simulink model "CCMVgaMotor"  (R2022b) (continued)
== system CCMVgaMotor/CCMVgaMotor_Runnable_sys == (continued)
      settings: EnsureOutportIsVirtual=on
  VentnMotSaveData_VentnMotSaveData_write : Outport  Port=15
      settings: EnsureOutportIsVirtual=on
  VentnMotSaveData2_VentnMotSaveData2_write : Outport  Port=16
      settings: EnsureOutportIsVirtual=on
  VentnMotSpdSet_VentnMotSpdSet_write : Outport  Port=17
      settings: EnsureOutportIsVirtual=on
  VentnMotSpdSet2_VentnMotSpdSet2_write : Outport  Port=18
      settings: EnsureOutportIsVirtual=on
  VentnMotStrtPson_VentnMotStrtPson_write : Outport  Port=19
      settings: EnsureOutportIsVirtual=on
  VentnMotStrtPson2_VentnMotStrtPson2_write : Outport  Port=20
      settings: EnsureOutportIsVirtual=on
  VentnMotTargtPson_VentnMotTargtPson_write : Outport  Port=21
      settings: EnsureOutportIsVirtual=on
  VentnMotTargtPson2_VentnMotTargtPson2_write : Outport  Port=22
      settings: EnsureOutportIsVirtual=on
  VentnMotUrgcPson_VentnMotUrgcPson_write : Outport  Port=23
      settings: EnsureOutportIsVirtual=on
  VentnMotUrgcPson2_VentnMotUrgcPson2_write : Outport  Port=24
      settings: EnsureOutportIsVirtual=on
  VentnMotUrgcUnlck_VentnMotUrgcUnlck_write : Outport  Port=25
      settings: EnsureOutportIsVirtual=on
  VentnMotUrgcUnlck2_VentnMotUrgcUnlck2_write : Outport  Port=26
      settings: EnsureOutportIsVirtual=on
  wires:
  CarCfgForClimaCtrl_CarCfgForAirClng_read/1 -> Terminator_1/1
  CarCfgForClimaCtrl_CarCfgForAirClnrAdv_read/1 -> Terminator_2/1
  CarCfgForClimaCtrl_CarCfgForAirFrag_read/1 -> Terminator_3/1
  CarCfgForClimaCtrl_CarCfgForAirMtrlSnsr_read/1 -> Terminator_4/1
  CarCfgForClimaCtrl_CarCfgForAirQly_read/1 -> Terminator_5/1
  CarCfgForClimaCtrl_CarCfgForAmntOfSeat_read/1 -> Terminator_6/1
  CarCfgForClimaCtrl_CarCfgForAuxWtrPmp_read/1 -> Terminator_7/1
  CarCfgForClimaCtrl_CarCfgForBypVlvInHvac_read/1 -> Terminator_8/1
  CarCfgForClimaCtrl_CarCfgForClsVlvInHvac_read/1 -> Terminator_9/1
  CarCfgForClimaCtrl_CarCfgForCmprAcTyp_read/1 -> Terminator_10/1
  CarCfgForClimaCtrl_CarCfgForCmptmtClng_read/1 -> Terminator_11/1
  CarCfgForClimaCtrl_CarCfgForElecVentAdjmt_read/1 -> Terminator_12/1
  CarCfgForClimaCtrl_CarCfgForFlcTyp_read/1 -> Terminator_13/1
  CarCfgForClimaCtrl_CarCfgForFoldCSD_read/1 -> Terminator_14/1
  CarCfgForClimaCtrl_CarCfgForFuTyp_read/1 -> Terminator_15/1
  CarCfgForClimaCtrl_CarCfgForGlassTyp_read/1 -> Terminator_16/1
  CarCfgForClimaCtrl_CarCfgForHeatPmp_read/1 -> Terminator_17/1
  CarCfgForClimaCtrl_CarCfgForHeatrDurgDrvg_read/1 -> Terminator_18/1
  CarCfgForClimaCtrl_CarCfgForHeatrTyp_read/1 -> Terminator_19/1
  CarCfgForClimaCtrl_CarCfgForHvacFilTyp_read/1 -> Terminator_20/1
  CarCfgForClimaCtrl_CarCfgForHvacFlrSnsrForT_read/1 -> Terminator_21/1
  CarCfgForClimaCtrl_CarCfgForHvacHeatrSnsrT_read/1 -> Terminator_22/1
  CarCfgForClimaCtrl_CarCfgForHvacRe_read/1 -> Terminator_23/1
  CarCfgForClimaCtrl_CarCfgForHvacTyp_read/1 -> Terminator_24/1
  CarCfgForClimaCtrl_CarCfgForLoVoltElecCfg_read/1 -> Terminator_25/1
  CarCfgForClimaCtrl_CarCfgForNavSysandIntnmap_read/1 -> Terminator_26/1
  CarCfgForClimaCtrl_CarCfgForNrOfDoor_read/1 -> Terminator_27/1
  CarCfgForClimaCtrl_CarCfgForPipeInFuTank_read/1 -> Terminator_28/1
  CarCfgForClimaCtrl_CarCfgForPreClng_read/1 -> Terminator_29/1
  CarCfgForClimaCtrl_CarCfgForPrkgCDSNotAvl_read/1 -> Terminator_30/1
  CarCfgForClimaCtrl_CarCfgForPrkgClima_read/1 -> Terminator_31/1
  CarCfgForClimaCtrl_CarCfgForPrpsnTyp_read/1 -> Terminator_32/1
  CarCfgForClimaCtrl_CarCfgForRemEngStrtPreCdn_read/1 -> Terminator_33/1
  CarCfgForClimaCtrl_CarCfgForRoofHatch_read/1 -> Terminator_34/1
  CarCfgForClimaCtrl_CarCfgForSeatHeatgCal_read/1 -> Terminator_35/1
  CarCfgForClimaCtrl_CarCfgForSeatHeatgForRowFirst_read/1 -> Terminator_36/1
  CarCfgForClimaCtrl_CarCfgForSeatHeatgForRowSec_read/1 -> Terminator_37/1
  CarCfgForClimaCtrl_CarCfgForSeatVentnForRowFirst_read/1 -> Terminator_38/1
  CarCfgForClimaCtrl_CarCfgForSeatVentnForRowSec_read/1 -> Terminator_39/1
  CarCfgForClimaCtrl_CarCfgForSecRowVentnCenter_read/1 -> Terminator_40/1
  CarCfgForClimaCtrl_CarCfgForSecRowVentnSide_read/1 -> Terminator_41/1
  CarCfgForClimaCtrl_CarCfgForSmallFlowCyc_read/1 -> Terminator_42/1
  CarCfgForClimaCtrl_CarCfgForSmkSnsr_read/1 -> Terminator_43/1
  CarCfgForClimaCtrl_CarCfgForSnsrForCmptmtT_read/1 -> Terminator_44/1
  CarCfgForClimaCtrl_CarCfgForSnsrHumPrsnt_read/1 -> Terminator_45/1
  CarCfgForClimaCtrl_CarCfgForSteerWhlHeatg_read/1 -> Terminator_46/1
  CarCfgForClimaCtrl_CarCfgForSteerWhlPosn_read/1 -> Terminator_47/1
  CarCfgForClimaCtrl_CarCfgForSunSnsr_read/1 -> Terminator_48/1
  CarCfgForClimaCtrl_CarCfgForTelmNodTyp_read/1 -> Terminator_49/1
  CarCfgForClimaCtrl_CarCfgForThermMngt_read/1 -> Terminator_50/1
  CarCfgForClimaCtrl_CarCfgForVehTyp_read/1 -> Terminator_51/1
  CarCfgForClimaCtrl_CarCfgForVgaMotorExistSts_read/1 -> Terminator_52/1
  CarCfgForClimaCtrl_CarCfgForWindHeatd_read/1 -> Terminator_53/1
  CarCfgForClimaCtrl_CarCfgForAutDemst_read/1 -> Terminator_54/1
  CarCfgForClimaCtrl_CarCfgForExtVentnClimaCtrl_read/1 -> Terminator_55/1
  CarCfgForClimaCtrl_CarCfgForStartDevice_read/1 -> Terminator_56/1
  HmiElecAirSwngReq_HmiElecAirSwngReq_read/1 -> Terminator_57/1
  LIN2ScheTableReq_Val_read/1 -> Terminator_58/1
  SysServCarCfgSts_SysServCarID_read/1 -> Terminator_59/1
  SysServCarCfgSts_SysServCCPAQSSts_read/1 -> Terminator_60/1
  SysServCarCfgSts_SysServCCPHEXSensorSts_read/1 -> Terminator_61/1
  SysServCarCfgSts_SysServCCPLin2AFUSts_read/1 -> Terminator_62/1
  SysServCarCfgSts_SysServCCPLin2AIUSts_read/1 -> Terminator_63/1
  SysServCarCfgSts_SysServCCPLin2CDSSts_read/1 -> Terminator_64/1
  SysServCarCfgSts_SysServCCPLin2PMSISts_read/1 -> Terminator_65/1
  SysServCarCfgSts_SysServCCPLin3VGASts_read/1 -> Terminator_66/1
  SysServCarCfgSts_SysServDUAirVentSnrSts_read/1 -> Terminator_67/1
  SysServCarCfgSts_SysServDUBlowerPwmSignSts_read/1 -> Terminator_68/1
  SysServCarCfgSts_SysServDUBlowerPwmSts_read/1 -> Terminator_69/1
  SysServCarCfgSts_SysServDUDCDefrostSts_read/1 -> Terminator_70/1
  SysServCarCfgSts_SysServDUDCModeSts_read/1 -> Terminator_71/1
  SysServCarCfgSts_SysServDUDCRecSts_read/1 -> Terminator_72/1
  SysServCarCfgSts_SysServDUDCSModeSts_read/1 -> Terminator_73/1
  SysServCarCfgSts_SysServDUDCSTLSts_read/1 -> Terminator_74/1
  SysServCarCfgSts_SysServDUDCTLSts_read/1 -> Terminator_75/1
  SysServCarCfgSts_SysServDUDCTRSts_read/1 -> Terminator_76/1
  SysServCarCfgSts_SysServDUFLFootSts_read/1 -> Terminator_77/1
  SysServCarCfgSts_SysServDUFLVentSts_read/1 -> Terminator_78/1
  SysServCarCfgSts_SysServDUFRFootSts_read/1 -> Terminator_79/1
  SysServCarCfgSts_SysServDUFRVentSts_read/1 -> Terminator_80/1
  SysServCarCfgSts_SysServDUIncarMotorSts_read/1 -> Terminator_81/1
  SysServCarCfgSts_SysServDULin1HBMFSts_read/1 -> Terminator_82/1
  SysServCarCfgSts_SysServDULin4HFAFSts_read/1 -> Terminator_83/1
  SysServCarCfgSts_SysServDUSFLFootSts_read/1 -> Terminator_84/1
  SysServCarCfgSts_SysServDUSFLVentSts_read/1 -> Terminator_85/1
  SysServCarCfgSts_SysServDUSFRFootSts_read/1 -> Terminator_86/1
  SysServCarCfgSts_SysServDUSFRVentSts_read/1 -> Terminator_87/1
  SysServCarCfgSts_SysServDUSnsrForFCmptmtTSts_read/1 -> Terminator_88/1
  SysServCarCfgSts_SysServDUSnsrForRair_read/1 -> Terminator_89/1
  SysServCarCfgSts_SysServDUSnsrForRCmptmtTSts_read/1 -> Terminator_90/1
  VehModMngtGlbSafe1_VehModMngtGlbSafe1_read/1 -> Terminator_91/1
  BatLoadBtrMSts_BatLoadBtrMSts_read/1 -> Terminator_92/1
  BatSysBtrMSts_BatSysBtrMSts_read/1 -> Terminator_93/1
  VgaMotSetPowerOnInit_PowerOnInit_read/1 -> Terminator_94/1
  CarCfgForClimaCtrl_CarCfgForBRAND_read/1 -> Terminator_95/1
  CarCfgForClimaCtrl_CarCfgForHeadRadar_read/1 -> Terminator_96/1
  CarCfgForClimaCtrl_CarCfgForInteriorViewCamera_read/1 -> Terminator_97/1
  CarCfgForClimaCtrl_CarCfgForLifeDetection_read/1 -> Terminator_98/1
  CarCfgForClimaCtrl_CarCfgForPowerSeats_read/1 -> Terminator_99/1
  CarCfgForClimaCtrl_CarCfgForPTCInFrontHVAC_read/1 -> Terminator_100/1
  CarCfgForClimaCtrl_CarCfgForVentLiftMotorExiSts_read/1 -> Terminator_101/1
  CarCfgForClimaCtrl_CarCfgForWadingAssistSystem_read/1 -> Terminator_102/1
  SbcPwrOn_SbcPwrOn_read/1 -> Terminator_103/1
  SbcWakeupSta_SbcWakeupSta_read/1 -> Terminator_104/1
  SysServCarCfgSts_SysServCCPBGMNum_read/1 -> Terminator_105/1
  SysServCarCfgSts_SysServCCPLin2AQSSts_read/1 -> Terminator_106/1
  SysServCarCfgSts_SysServDUBlowerPwmSignTypeSts_read/1 -> Terminator_107/1
  SysServCarCfgSts_SysServDUDCFLMdeSts_read/1 -> Terminator_108/1
  SysServCarCfgSts_SysServDUReBlowerPwmSignSts_read/1 -> Terminator_109/1
  SysServCarCfgSts_SysServDUReBlowerPwmSts_read/1 -> Terminator_110/1
  SysServCarCfgSts_SysServLowSideRETXVSts_read/1 -> Terminator_111/1
  VentnActr01ActPson_VentnActr01ActPson_read/1 -> Terminator_112/1
  VentnActr01ActPson_VentnActr01ActPson_IsUpdated_read/1 -> Terminator_113/1
  VentnActr01BlckInd_VentnActr01BlckInd_read/1 -> Terminator_114/1
  VentnActr01BlckSts_VentnActr01BlckSts_read/1 -> Terminator_115/1
  VentnActr01BusAdr_VentnActr01BusAdr_read/1 -> Terminator_116/1
  VentnActr01CoilSts_VentnActr01CoilSts_read/1 -> Terminator_117/1
  VentnActr01DrvDir_VentnActr01DrvDir_read/1 -> Terminator_118/1
  VentnActr01ElecErr_VentnActr01ElecErr_read/1 -> Terminator_119/1
  VentnActr01Mode_VentnActr01Mode_read/1 -> Terminator_120/1
  VentnActr01MovDir_VentnActr01MovDir_read/1 -> Terminator_121/1
  VentnActr01OvrTempErr_VentnActr01OvrTempErr_read/1 -> Terminator_122/1
  VentnActr01PsonSts_VentnActr01PsonSts_read/1 -> Terminator_123/1
  VentnActr01ResetSts_VentnActr01ResetSts_read/1 -> Terminator_124/1
  VentnActr01SpclFct_VentnActr01SpclFct_read/1 -> Terminator_125/1
  VentnActr01SpdSts_VentnActr01SpdSts_read/1 -> Terminator_126/1
  VentnActr01TrqSts_VentnActr01TrqSts_read/1 -> Terminator_127/1
  VentnActr01UrgcExist_VentnActr01UrgcExist_read/1 -> Terminator_128/1
  VentnActr01UrgcPson_VentnActr01UrgcPson_read/1 -> Terminator_129/1
  VentnActr01UrgcSts_VentnActr01UrgcSts_read/1 -> Terminator_130/1
  VentnActr01VoltErr_VentnActr01VoltErr_read/1 -> Terminator_131/1
  VentnActr02ActPson_VentnActr02ActPson_read/1 -> Terminator_132/1
  VentnActr02ActPson_VentnActr02ActPson_IsUpdated_read/1 -> Terminator_133/1
  VentnActr02BlckInd_VentnActr02BlckInd_read/1 -> Terminator_134/1
  VentnActr02BlckSts_VentnActr02BlckSts_read/1 -> Terminator_135/1
  VentnActr02BusAdr_VentnActr02BusAdr_read/1 -> Terminator_136/1
  VentnActr02CoilSts_VentnActr02CoilSts_read/1 -> Terminator_137/1
  VentnActr02DrvDir_VentnActr02DrvDir_read/1 -> Terminator_138/1
  VentnActr02ElecErr_VentnActr02ElecErr_read/1 -> Terminator_139/1
  VentnActr02Mode_VentnActr02Mode_read/1 -> Terminator_140/1
  VentnActr02MovDir_VentnActr02MovDir_read/1 -> Terminator_141/1
  VentnActr02OvrTempErr_VentnActr02OvrTempErr_read/1 -> Terminator_142/1
  VentnActr02PsonSts_VentnActr02PsonSts_read/1 -> Terminator_143/1
  VentnActr02ResetSts_VentnActr02ResetSts_read/1 -> Terminator_144/1
  VentnActr02SpclFct_VentnActr02SpclFct_read/1 -> Terminator_145/1
  VentnActr02SpdSts_VentnActr02SpdSts_read/1 -> Terminator_146/1
  VentnActr02TrqSts_VentnActr02TrqSts_read/1 -> Terminator_147/1
  VentnActr02UrgcExist_VentnActr02UrgcExist_read/1 -> Terminator_148/1
  VentnActr02UrgcPson_VentnActr02UrgcPson_read/1 -> Terminator_149/1
  VentnActr02UrgcSts_VentnActr02UrgcSts_read/1 -> Terminator_150/1
  VentnActr02VoltErr_VentnActr02VoltErr_read/1 -> Terminator_151/1
  VentnActr03ActPson_VentnActr03ActPson_read/1 -> Terminator_152/1
  VentnActr03ActPson_VentnActr03ActPson_IsUpdated_read/1 -> Terminator_153/1
  VentnActr03BlckInd_VentnActr03BlckInd_read/1 -> Terminator_154/1
  VentnActr03BlckSts_VentnActr03BlckSts_read/1 -> Terminator_155/1
  VentnActr03BusAdr_VentnActr03BusAdr_read/1 -> Terminator_156/1
  VentnActr03CoilSts_VentnActr03CoilSts_read/1 -> Terminator_157/1
  VentnActr03DrvDir_VentnActr03DrvDir_read/1 -> Terminator_158/1
  VentnActr03ElecErr_VentnActr03ElecErr_read/1 -> Terminator_159/1
  VentnActr03Mode_VentnActr03Mode_read/1 -> Terminator_160/1
  VentnActr03MovDir_VentnActr03MovDir_read/1 -> Terminator_161/1
  VentnActr03OvrTempErr_VentnActr03OvrTempErr_read/1 -> Terminator_162/1
  VentnActr03PsonSts_VentnActr03PsonSts_read/1 -> Terminator_163/1
  VentnActr03ResetSts_VentnActr03ResetSts_read/1 -> Terminator_164/1
  VentnActr03SpclFct_VentnActr03SpclFct_read/1 -> Terminator_165/1
  VentnActr03SpdSts_VentnActr03SpdSts_read/1 -> Terminator_166/1
  VentnActr03TrqSts_VentnActr03TrqSts_read/1 -> Terminator_167/1
  VentnActr03UrgcExist_VentnActr03UrgcExist_read/1 -> Terminator_168/1
  VentnActr03UrgcPson_VentnActr03UrgcPson_read/1 -> Terminator_169/1
  VentnActr03UrgcSts_VentnActr03UrgcSts_read/1 -> Terminator_170/1
  VentnActr03VoltErr_VentnActr03VoltErr_read/1 -> Terminator_171/1
  VentnActr04ActPson_VentnActr04ActPson_read/1 -> Terminator_172/1
  VentnActr04ActPson_VentnActr04ActPson_IsUpdated_read/1 -> Terminator_173/1
  VentnActr04BlckInd_VentnActr04BlckInd_read/1 -> Terminator_174/1
  VentnActr04BlckSts_VentnActr04BlckSts_read/1 -> Terminator_175/1
  VentnActr04BusAdr_VentnActr04BusAdr_read/1 -> Terminator_176/1
  VentnActr04CoilSts_VentnActr04CoilSts_read/1 -> Terminator_177/1
  VentnActr04DrvDir_VentnActr04DrvDir_read/1 -> Terminator_178/1
  VentnActr04ElecErr_VentnActr04ElecErr_read/1 -> Terminator_179/1
  VentnActr04Mode_VentnActr04Mode_read/1 -> Terminator_180/1
  VentnActr04MovDir_VentnActr04MovDir_read/1 -> Terminator_181/1
  VentnActr04OvrTempErr_VentnActr04OvrTempErr_read/1 -> Terminator_182/1
  VentnActr04PsonSts_VentnActr04PsonSts_read/1 -> Terminator_183/1
  VentnActr04ResetSts_VentnActr04ResetSts_read/1 -> Terminator_184/1
  VentnActr04SpclFct_VentnActr04SpclFct_read/1 -> Terminator_185/1
  VentnActr04SpdSts_VentnActr04SpdSts_read/1 -> Terminator_186/1
  VentnActr04TrqSts_VentnActr04TrqSts_read/1 -> Terminator_187/1
  VentnActr04UrgcExist_VentnActr04UrgcExist_read/1 -> Terminator_188/1
  VentnActr04UrgcPson_VentnActr04UrgcPson_read/1 -> Terminator_189/1
  VentnActr04UrgcSts_VentnActr04UrgcSts_read/1 -> Terminator_190/1
  VentnActr04VoltErr_VentnActr04VoltErr_read/1 -> Terminator_191/1
  VentnActr05ActPson_VentnActr05ActPson_read/1 -> Terminator_192/1
  VentnActr05ActPson_VentnActr05ActPson_IsUpdated_read/1 -> Terminator_193/1
  VentnActr05BlckInd_VentnActr05BlckInd_read/1 -> Terminator_194/1
  VentnActr05BlckSts_VentnActr05BlckSts_read/1 -> Terminator_195/1
  VentnActr05BusAdr_VentnActr05BusAdr_read/1 -> Terminator_196/1
  VentnActr05CoilSts_VentnActr05CoilSts_read/1 -> Terminator_197/1
  VentnActr05DrvDir_VentnActr05DrvDir_read/1 -> Terminator_198/1
  VentnActr05ElecErr_VentnActr05ElecErr_read/1 -> Terminator_199/1
  VentnActr05Mode_VentnActr05Mode_read/1 -> Terminator_200/1
  VentnActr05MovDir_VentnActr05MovDir_read/1 -> Terminator_201/1
  VentnActr05OvrTempErr_VentnActr05OvrTempErr_read/1 -> Terminator_202/1
  VentnActr05PsonSts_VentnActr05PsonSts_read/1 -> Terminator_203/1
  VentnActr05ResetSts_VentnActr05ResetSts_read/1 -> Terminator_204/1
  VentnActr05SpclFct_VentnActr05SpclFct_read/1 -> Terminator_205/1
  VentnActr05SpdSts_VentnActr05SpdSts_read/1 -> Terminator_206/1
  VentnActr05TrqSts_VentnActr05TrqSts_read/1 -> Terminator_207/1
  VentnActr05UrgcExist_VentnActr05UrgcExist_read/1 -> Terminator_208/1
  VentnActr05UrgcPson_VentnActr05UrgcPson_read/1 -> Terminator_209/1
  VentnActr05UrgcSts_VentnActr05UrgcSts_read/1 -> Terminator_210/1
  VentnActr05VoltErr_VentnActr05VoltErr_read/1 -> Terminator_211/1
  VentnActr06ActPson_VentnActr06ActPson_read/1 -> Terminator_212/1
  VentnActr06ActPson_VentnActr06ActPson_IsUpdated_read/1 -> Terminator_213/1
  VentnActr06BlckInd_VentnActr06BlckInd_read/1 -> Terminator_214/1
  VentnActr06BlckSts_VentnActr06BlckSts_read/1 -> Terminator_215/1
  VentnActr06BusAdr_VentnActr06BusAdr_read/1 -> Terminator_216/1
  VentnActr06CoilSts_VentnActr06CoilSts_read/1 -> Terminator_217/1
  VentnActr06DrvDir_VentnActr06DrvDir_read/1 -> Terminator_218/1
  VentnActr06ElecErr_VentnActr06ElecErr_read/1 -> Terminator_219/1
  VentnActr06Mode_VentnActr06Mode_read/1 -> Terminator_220/1
  VentnActr06MovDir_VentnActr06MovDir_read/1 -> Terminator_221/1
  VentnActr06OvrTempErr_VentnActr06OvrTempErr_read/1 -> Terminator_222/1
  VentnActr06PsonSts_VentnActr06PsonSts_read/1 -> Terminator_223/1
  VentnActr06ResetSts_VentnActr06ResetSts_read/1 -> Terminator_224/1
  VentnActr06SpclFct_VentnActr06SpclFct_read/1 -> Terminator_225/1
  VentnActr06SpdSts_VentnActr06SpdSts_read/1 -> Terminator_226/1
  VentnActr06TrqSts_VentnActr06TrqSts_read/1 -> Terminator_227/1
  VentnActr06UrgcExist_VentnActr06UrgcExist_read/1 -> Terminator_228/1
  VentnActr06UrgcPson_VentnActr06UrgcPson_read/1 -> Terminator_229/1
  VentnActr06UrgcSts_VentnActr06UrgcSts_read/1 -> Terminator_230/1
  VentnActr06VoltErr_VentnActr06VoltErr_read/1 -> Terminator_231/1
  VentnActr07ActPson_VentnActr07ActPson_read/1 -> Terminator_232/1
  VentnActr07ActPson_VentnActr07ActPson_IsUpdated_read/1 -> Terminator_233/1
  VentnActr07BlckInd_VentnActr07BlckInd_read/1 -> Terminator_234/1
  VentnActr07BlckSts_VentnActr07BlckSts_read/1 -> Terminator_235/1
  VentnActr07BusAdr_VentnActr07BusAdr_read/1 -> Terminator_236/1
  VentnActr07CoilSts_VentnActr07CoilSts_read/1 -> Terminator_237/1
  VentnActr07DrvDir_VentnActr07DrvDir_read/1 -> Terminator_238/1
  VentnActr07ElecErr_VentnActr07ElecErr_read/1 -> Terminator_239/1
  VentnActr07Mode_VentnActr07Mode_read/1 -> Terminator_240/1
  VentnActr07MovDir_VentnActr07MovDir_read/1 -> Terminator_241/1
  VentnActr07OvrTempErr_VentnActr07OvrTempErr_read/1 -> Terminator_242/1
  VentnActr07PsonSts_VentnActr07PsonSts_read/1 -> Terminator_243/1
  VentnActr07ResetSts_VentnActr07ResetSts_read/1 -> Terminator_244/1
  VentnActr07SpclFct_VentnActr07SpclFct_read/1 -> Terminator_245/1
  VentnActr07SpdSts_VentnActr07SpdSts_read/1 -> Terminator_246/1
  VentnActr07TrqSts_VentnActr07TrqSts_read/1 -> Terminator_247/1
  VentnActr07UrgcExist_VentnActr07UrgcExist_read/1 -> Terminator_248/1
  VentnActr07UrgcPson_VentnActr07UrgcPson_read/1 -> Terminator_249/1
  VentnActr07UrgcSts_VentnActr07UrgcSts_read/1 -> Terminator_250/1
  VentnActr07VoltErr_VentnActr07VoltErr_read/1 -> Terminator_251/1
  VentnActr08ActPson_VentnActr08ActPson_read/1 -> Terminator_252/1
  VentnActr08ActPson_VentnActr08ActPson_IsUpdated_read/1 -> Terminator_253/1
  VentnActr08BlckInd_VentnActr08BlckInd_read/1 -> Terminator_254/1
  VentnActr08BlckSts_VentnActr08BlckSts_read/1 -> Terminator_255/1
  VentnActr08BusAdr_VentnActr08BusAdr_read/1 -> Terminator_256/1
  VentnActr08CoilSts_VentnActr08CoilSts_read/1 -> Terminator_257/1
  VentnActr08DrvDir_VentnActr08DrvDir_read/1 -> Terminator_258/1
  VentnActr08ElecErr_VentnActr08ElecErr_read/1 -> Terminator_259/1
  VentnActr08Mode_VentnActr08Mode_read/1 -> Terminator_260/1
  VentnActr08MovDir_VentnActr08MovDir_read/1 -> Terminator_261/1
  VentnActr08OvrTempErr_VentnActr08OvrTempErr_read/1 -> Terminator_262/1
  VentnActr08PsonSts_VentnActr08PsonSts_read/1 -> Terminator_263/1
  VentnActr08ResetSts_VentnActr08ResetSts_read/1 -> Terminator_264/1
  VentnActr08SpclFct_VentnActr08SpclFct_read/1 -> Terminator_265/1
  VentnActr08SpdSts_VentnActr08SpdSts_read/1 -> Terminator_266/1
  VentnActr08TrqSts_VentnActr08TrqSts_read/1 -> Terminator_267/1
  VentnActr08UrgcExist_VentnActr08UrgcExist_read/1 -> Terminator_268/1
  VentnActr08UrgcPson_VentnActr08UrgcPson_read/1 -> Terminator_269/1
  VentnActr08UrgcSts_VentnActr08UrgcSts_read/1 -> Terminator_270/1
  VentnActr08VoltErr_VentnActr08VoltErr_read/1 -> Terminator_271/1
  VentnActr09ActPson_VentnActr09ActPson_read/1 -> Terminator_272/1
  VentnActr09ActPson_VentnActr09ActPson_IsUpdated_read/1 -> Terminator_273/1
  VentnActr09BlckInd_VentnActr09BlckInd_read/1 -> Terminator_274/1
  VentnActr09BlckSts_VentnActr09BlckSts_read/1 -> Terminator_275/1
  VentnActr09BusAdr_VentnActr09BusAdr_read/1 -> Terminator_276/1
  VentnActr09CoilSts_VentnActr09CoilSts_read/1 -> Terminator_277/1
  VentnActr09DrvDir_VentnActr09DrvDir_read/1 -> Terminator_278/1
  VentnActr09ElecErr_VentnActr09ElecErr_read/1 -> Terminator_279/1
  VentnActr09Mode_VentnActr09Mode_read/1 -> Terminator_280/1
  VentnActr09MovDir_VentnActr09MovDir_read/1 -> Terminator_281/1
  VentnActr09OvrTempErr_VentnActr09OvrTempErr_read/1 -> Terminator_282/1
  VentnActr09PsonSts_VentnActr09PsonSts_read/1 -> Terminator_283/1
  VentnActr09ResetSts_VentnActr09ResetSts_read/1 -> Terminator_284/1
  VentnActr09SpclFct_VentnActr09SpclFct_read/1 -> Terminator_285/1
  VentnActr09SpdSts_VentnActr09SpdSts_read/1 -> Terminator_286/1
  VentnActr09TrqSts_VentnActr09TrqSts_read/1 -> Terminator_287/1
  VentnActr09UrgcExist_VentnActr09UrgcExist_read/1 -> Terminator_288/1
  VentnActr09UrgcPson_VentnActr09UrgcPson_read/1 -> Terminator_289/1
  VentnActr09UrgcSts_VentnActr09UrgcSts_read/1 -> Terminator_290/1
  VentnActr09VoltErr_VentnActr09VoltErr_read/1 -> Terminator_291/1
  VentnActr10ActPson_VentnActr10ActPson_read/1 -> Terminator_292/1
  VentnActr10ActPson_VentnActr10ActPson_IsUpdated_read/1 -> Terminator_293/1
  VentnActr10BlckInd_VentnActr10BlckInd_read/1 -> Terminator_294/1
  VentnActr10BlckSts_VentnActr10BlckSts_read/1 -> Terminator_295/1
  VentnActr10BusAdr_VentnActr10BusAdr_read/1 -> Terminator_296/1
  VentnActr10CoilSts_VentnActr10CoilSts_read/1 -> Terminator_297/1
  VentnActr10DrvDir_VentnActr10DrvDir_read/1 -> Terminator_298/1
  VentnActr10ElecErr_VentnActr10ElecErr_read/1 -> Terminator_299/1
  VentnActr10Mode_VentnActr10Mode_read/1 -> Terminator_300/1
  VentnActr10MovDir_VentnActr10MovDir_read/1 -> Terminator_301/1
  VentnActr10OvrTempErr_VentnActr10OvrTempErr_read/1 -> Terminator_302/1
  VentnActr10PsonSts_VentnActr10PsonSts_read/1 -> Terminator_303/1
  VentnActr10ResetSts_VentnActr10ResetSts_read/1 -> Terminator_304/1
  VentnActr10SpclFct_VentnActr10SpclFct_read/1 -> Terminator_305/1
  VentnActr10SpdSts_VentnActr10SpdSts_read/1 -> Terminator_306/1
  VentnActr10TrqSts_VentnActr10TrqSts_read/1 -> Terminator_307/1
  VentnActr10UrgcExist_VentnActr10UrgcExist_read/1 -> Terminator_308/1
  VentnActr10UrgcPson_VentnActr10UrgcPson_read/1 -> Terminator_309/1
  VentnActr10UrgcSts_VentnActr10UrgcSts_read/1 -> Terminator_310/1
  VentnActr10VoltErr_VentnActr10VoltErr_read/1 -> Terminator_311/1
  VentnActr11ActPson_VentnActr11ActPson_read/1 -> Terminator_312/1
  VentnActr11ActPson_VentnActr11ActPson_IsUpdated_read/1 -> Terminator_313/1
  VentnActr11BlckInd_VentnActr11BlckInd_read/1 -> Terminator_314/1
  VentnActr11BlckSts_VentnActr11BlckSts_read/1 -> Terminator_315/1
  VentnActr11BusAdr_VentnActr11BusAdr_read/1 -> Terminator_316/1
  VentnActr11CoilSts_VentnActr11CoilSts_read/1 -> Terminator_317/1
  VentnActr11DrvDir_VentnActr11DrvDir_read/1 -> Terminator_318/1
  VentnActr11ElecErr_VentnActr11ElecErr_read/1 -> Terminator_319/1
  VentnActr11Mode_VentnActr11Mode_read/1 -> Terminator_320/1
  VentnActr11MovDir_VentnActr11MovDir_read/1 -> Terminator_321/1
  VentnActr11OvrTempErr_VentnActr11OvrTempErr_read/1 -> Terminator_322/1
  VentnActr11PsonSts_VentnActr11PsonSts_read/1 -> Terminator_323/1
  VentnActr11ResetSts_VentnActr11ResetSts_read/1 -> Terminator_324/1
  VentnActr11SpclFct_VentnActr11SpclFct_read/1 -> Terminator_325/1
  VentnActr11SpdSts_VentnActr11SpdSts_read/1 -> Terminator_326/1
  VentnActr11TrqSts_VentnActr11TrqSts_read/1 -> Terminator_327/1
  VentnActr11UrgcExist_VentnActr11UrgcExist_read/1 -> Terminator_328/1
  VentnActr11UrgcPson_VentnActr11UrgcPson_read/1 -> Terminator_329/1
  VentnActr11UrgcSts_VentnActr11UrgcSts_read/1 -> Terminator_330/1
  VentnActr11VoltErr_VentnActr11VoltErr_read/1 -> Terminator_331/1
  VentnActr12ActPson_VentnActr12ActPson_read/1 -> Terminator_332/1
  VentnActr12ActPson_VentnActr12ActPson_IsUpdated_read/1 -> Terminator_333/1
  VentnActr12BlckInd_VentnActr12BlckInd_read/1 -> Terminator_334/1
  VentnActr12BlckSts_VentnActr12BlckSts_read/1 -> Terminator_335/1
  VentnActr12BusAdr_VentnActr12BusAdr_read/1 -> Terminator_336/1
  VentnActr12CoilSts_VentnActr12CoilSts_read/1 -> Terminator_337/1
  VentnActr12DrvDir_VentnActr12DrvDir_read/1 -> Terminator_338/1
  VentnActr12ElecErr_VentnActr12ElecErr_read/1 -> Terminator_339/1
  VentnActr12Mode_VentnActr12Mode_read/1 -> Terminator_340/1
  VentnActr12MovDir_VentnActr12MovDir_read/1 -> Terminator_341/1
  VentnActr12OvrTempErr_VentnActr12OvrTempErr_read/1 -> Terminator_342/1
  VentnActr12PsonSts_VentnActr12PsonSts_read/1 -> Terminator_343/1
  VentnActr12ResetSts_VentnActr12ResetSts_read/1 -> Terminator_344/1
  VentnActr12SpclFct_VentnActr12SpclFct_read/1 -> Terminator_345/1
  VentnActr12SpdSts_VentnActr12SpdSts_read/1 -> Terminator_346/1
  VentnActr12TrqSts_VentnActr12TrqSts_read/1 -> Terminator_347/1
  VentnActr12UrgcExist_VentnActr12UrgcExist_read/1 -> Terminator_348/1
  VentnActr12UrgcPson_VentnActr12UrgcPson_read/1 -> Terminator_349/1
  VentnActr12UrgcSts_VentnActr12UrgcSts_read/1 -> Terminator_350/1
  VentnActr12VoltErr_VentnActr12VoltErr_read/1 -> Terminator_351/1
  VentnActr13ActPson_VentnActr13ActPson_read/1 -> Terminator_352/1
  VentnActr13ActPson_VentnActr13ActPson_IsUpdated_read/1 -> Terminator_353/1
  VentnActr13BlckInd_VentnActr13BlckInd_read/1 -> Terminator_354/1
  VentnActr13BlckSts_VentnActr13BlckSts_read/1 -> Terminator_355/1
  VentnActr13BusAdr_VentnActr13BusAdr_read/1 -> Terminator_356/1
  VentnActr13CoilSts_VentnActr13CoilSts_read/1 -> Terminator_357/1
  VentnActr13DrvDir_VentnActr13DrvDir_read/1 -> Terminator_358/1
  VentnActr13ElecErr_VentnActr13ElecErr_read/1 -> Terminator_359/1
  VentnActr13Mode_VentnActr13Mode_read/1 -> Terminator_360/1
  VentnActr13MovDir_VentnActr13MovDir_read/1 -> Terminator_361/1
  VentnActr13OvrTempErr_VentnActr13OvrTempErr_read/1 -> Terminator_362/1
  VentnActr13PsonSts_VentnActr13PsonSts_read/1 -> Terminator_363/1
  VentnActr13ResetSts_VentnActr13ResetSts_read/1 -> Terminator_364/1
  VentnActr13SpclFct_VentnActr13SpclFct_read/1 -> Terminator_365/1
  VentnActr13SpdSts_VentnActr13SpdSts_read/1 -> Terminator_366/1
  VentnActr13TrqSts_VentnActr13TrqSts_read/1 -> Terminator_367/1
  VentnActr13UrgcExist_VentnActr13UrgcExist_read/1 -> Terminator_368/1
  VentnActr13UrgcPson_VentnActr13UrgcPson_read/1 -> Terminator_369/1
  VentnActr13UrgcSts_VentnActr13UrgcSts_read/1 -> Terminator_370/1
  VentnActr13VoltErr_VentnActr13VoltErr_read/1 -> Terminator_371/1
  VentnActr14ActPson_VentnActr14ActPson_read/1 -> Terminator_372/1
  VentnActr14ActPson_VentnActr14ActPson_IsUpdated_read/1 -> Terminator_373/1
  VentnActr14BlckInd_VentnActr14BlckInd_read/1 -> Terminator_374/1
  VentnActr14BlckSts_VentnActr14BlckSts_read/1 -> Terminator_375/1
  VentnActr14BusAdr_VentnActr14BusAdr_read/1 -> Terminator_376/1
  VentnActr14CoilSts_VentnActr14CoilSts_read/1 -> Terminator_377/1
  VentnActr14DrvDir_VentnActr14DrvDir_read/1 -> Terminator_378/1
  VentnActr14ElecErr_VentnActr14ElecErr_read/1 -> Terminator_379/1
  VentnActr14Mode_VentnActr14Mode_read/1 -> Terminator_380/1
  VentnActr14MovDir_VentnActr14MovDir_read/1 -> Terminator_381/1
  VentnActr14OvrTempErr_VentnActr14OvrTempErr_read/1 -> Terminator_382/1
  VentnActr14PsonSts_VentnActr14PsonSts_read/1 -> Terminator_383/1
  VentnActr14ResetSts_VentnActr14ResetSts_read/1 -> Terminator_384/1
  VentnActr14SpclFct_VentnActr14SpclFct_read/1 -> Terminator_385/1
  VentnActr14SpdSts_VentnActr14SpdSts_read/1 -> Terminator_386/1
  VentnActr14TrqSts_VentnActr14TrqSts_read/1 -> Terminator_387/1
  VentnActr14UrgcExist_VentnActr14UrgcExist_read/1 -> Terminator_388/1
  VentnActr14UrgcPson_VentnActr14UrgcPson_read/1 -> Terminator_389/1
  VentnActr14UrgcSts_VentnActr14UrgcSts_read/1 -> Terminator_390/1
  VentnActr14VoltErr_VentnActr14VoltErr_read/1 -> Terminator_391/1
  VentnActr15ActPson_VentnActr15ActPson_read/1 -> Terminator_392/1
  VentnActr15ActPson_VentnActr15ActPson_IsUpdated_read/1 -> Terminator_393/1
  VentnActr15BlckInd_VentnActr15BlckInd_read/1 -> Terminator_394/1
  VentnActr15BlckSts_VentnActr15BlckSts_read/1 -> Terminator_395/1
  VentnActr15BusAdr_VentnActr15BusAdr_read/1 -> Terminator_396/1
  VentnActr15CoilSts_VentnActr15CoilSts_read/1 -> Terminator_397/1
  VentnActr15DrvDir_VentnActr15DrvDir_read/1 -> Terminator_398/1
  VentnActr15ElecErr_VentnActr15ElecErr_read/1 -> Terminator_399/1
  VentnActr15Mode_VentnActr15Mode_read/1 -> Terminator_400/1
  VentnActr15MovDir_VentnActr15MovDir_read/1 -> Terminator_401/1
  VentnActr15OvrTempErr_VentnActr15OvrTempErr_read/1 -> Terminator_402/1
  VentnActr15PsonSts_VentnActr15PsonSts_read/1 -> Terminator_403/1
  VentnActr15ResetSts_VentnActr15ResetSts_read/1 -> Terminator_404/1
  VentnActr15SpclFct_VentnActr15SpclFct_read/1 -> Terminator_405/1
  VentnActr15SpdSts_VentnActr15SpdSts_read/1 -> Terminator_406/1
  VentnActr15TrqSts_VentnActr15TrqSts_read/1 -> Terminator_407/1
  VentnActr15UrgcExist_VentnActr15UrgcExist_read/1 -> Terminator_408/1
  VentnActr15UrgcPson_VentnActr15UrgcPson_read/1 -> Terminator_409/1
  VentnActr15UrgcSts_VentnActr15UrgcSts_read/1 -> Terminator_410/1
  VentnActr15VoltErr_VentnActr15VoltErr_read/1 -> Terminator_411/1
  VentnActr16ActPson_VentnActr16ActPson_read/1 -> Terminator_412/1
  VentnActr16BlckInd_VentnActr16BlckInd_read/1 -> Terminator_413/1
  VentnActr16BlckSts_VentnActr16BlckSts_read/1 -> Terminator_414/1
  VentnActr16BusAdr_VentnActr16BusAdr_read/1 -> Terminator_415/1
  VentnActr16CoilSts_VentnActr16CoilSts_read/1 -> Terminator_416/1
  VentnActr16DrvDir_VentnActr16DrvDir_read/1 -> Terminator_417/1
  VentnActr16ElecErr_VentnActr16ElecErr_read/1 -> Terminator_418/1
  VentnActr16Mode_VentnActr16Mode_read/1 -> Terminator_419/1
  VentnActr16MovDir_VentnActr16MovDir_read/1 -> Terminator_420/1
  VentnActr16OvrTempErr_VentnActr16OvrTempErr_read/1 -> Terminator_421/1
  VentnActr16PsonSts_VentnActr16PsonSts_read/1 -> Terminator_422/1
  VentnActr16ResetSts_VentnActr16ResetSts_read/1 -> Terminator_423/1
  VentnActr16SpclFct_VentnActr16SpclFct_read/1 -> Terminator_424/1
  VentnActr16SpdSts_VentnActr16SpdSts_read/1 -> Terminator_425/1
  VentnActr16TrqSts_VentnActr16TrqSts_read/1 -> Terminator_426/1
  VentnActr16UrgcExist_VentnActr16UrgcExist_read/1 -> Terminator_427/1
  VentnActr16UrgcPson_VentnActr16UrgcPson_read/1 -> Terminator_428/1
  VentnActr16UrgcSts_VentnActr16UrgcSts_read/1 -> Terminator_429/1
  VentnActr16VoltErr_VentnActr16VoltErr_read/1 -> Terminator_430/1
  VentnActr17ActPson_VentnActr17ActPson_read/1 -> Terminator_431/1
  VentnActr17ActPson_VentnActr17ActPson_IsUpdated_read/1 -> Terminator_432/1
  VentnActr17BlckInd_VentnActr17BlckInd_read/1 -> Terminator_433/1
  VentnActr17BlckSts_VentnActr17BlckSts_read/1 -> Terminator_434/1
  VentnActr17BusAdr_VentnActr17BusAdr_read/1 -> Terminator_435/1
  VentnActr17CoilSts_VentnActr17CoilSts_read/1 -> Terminator_436/1
  VentnActr17DrvDir_VentnActr17DrvDir_read/1 -> Terminator_437/1
  VentnActr17ElecErr_VentnActr17ElecErr_read/1 -> Terminator_438/1
  VentnActr17Mode_VentnActr17Mode_read/1 -> Terminator_439/1
  VentnActr17MovDir_VentnActr17MovDir_read/1 -> Terminator_440/1
  VentnActr17OvrTempErr_VentnActr17OvrTempErr_read/1 -> Terminator_441/1
  VentnActr17PsonSts_VentnActr17PsonSts_read/1 -> Terminator_442/1
  VentnActr17ResetSts_VentnActr17ResetSts_read/1 -> Terminator_443/1
  VentnActr17SpclFct_VentnActr17SpclFct_read/1 -> Terminator_444/1
  VentnActr17SpdSts_VentnActr17SpdSts_read/1 -> Terminator_445/1
  VentnActr17TrqSts_VentnActr17TrqSts_read/1 -> Terminator_446/1
  VentnActr17UrgcExist_VentnActr17UrgcExist_read/1 -> Terminator_447/1
  VentnActr17UrgcPson_VentnActr17UrgcPson_read/1 -> Terminator_448/1
  VentnActr17UrgcSts_VentnActr17UrgcSts_read/1 -> Terminator_449/1
  VentnActr17VoltErr_VentnActr17VoltErr_read/1 -> Terminator_450/1
  VentnActr18ActPson_VentnActr18ActPson_read/1 -> Terminator_451/1
  VentnActr18BlckInd_VentnActr18BlckInd_read/1 -> Terminator_452/1
  VentnActr18BlckSts_VentnActr18BlckSts_read/1 -> Terminator_453/1
  VentnActr18BusAdr_VentnActr18BusAdr_read/1 -> Terminator_454/1
  VentnActr18CoilSts_VentnActr18CoilSts_read/1 -> Terminator_455/1
  VentnActr18DrvDir_VentnActr18DrvDir_read/1 -> Terminator_456/1
  VentnActr18ElecErr_VentnActr18ElecErr_read/1 -> Terminator_457/1
  VentnActr18Mode_VentnActr18Mode_read/1 -> Terminator_458/1
  VentnActr18MovDir_VentnActr18MovDir_read/1 -> Terminator_459/1
  VentnActr18OvrTempErr_VentnActr18OvrTempErr_read/1 -> Terminator_460/1
  VentnActr18PsonSts_VentnActr18PsonSts_read/1 -> Terminator_461/1
  VentnActr18ResetSts_VentnActr18ResetSts_read/1 -> Terminator_462/1
  VentnActr18SpclFct_VentnActr18SpclFct_read/1 -> Terminator_463/1
  VentnActr18SpdSts_VentnActr18SpdSts_read/1 -> Terminator_464/1
  VentnActr18TrqSts_VentnActr18TrqSts_read/1 -> Terminator_465/1
  VentnActr18UrgcExist_VentnActr18UrgcExist_read/1 -> Terminator_466/1
  VentnActr18UrgcPson_VentnActr18UrgcPson_read/1 -> Terminator_467/1
  VentnActr18UrgcSts_VentnActr18UrgcSts_read/1 -> Terminator_468/1
  VentnActr18VoltErr_VentnActr18VoltErr_read/1 -> Terminator_469/1
  VentnActr19ActPson_VentnActr19ActPson_read/1 -> Terminator_470/1
  VentnActr19ActPson_VentnActr19ActPson_IsUpdated_read/1 -> Terminator_471/1
  VentnActr19BlckInd_VentnActr19BlckInd_read/1 -> Terminator_472/1
  VentnActr19BlckSts_VentnActr19BlckSts_read/1 -> Terminator_473/1
  VentnActr19BusAdr_VentnActr19BusAdr_read/1 -> Terminator_474/1
  VentnActr19CoilSts_VentnActr19CoilSts_read/1 -> Terminator_475/1
  VentnActr19DrvDir_VentnActr19DrvDir_read/1 -> Terminator_476/1
  VentnActr19ElecErr_VentnActr19ElecErr_read/1 -> Terminator_477/1
  VentnActr19Mode_VentnActr19Mode_read/1 -> Terminator_478/1
  VentnActr19MovDir_VentnActr19MovDir_read/1 -> Terminator_479/1
  VentnActr19OvrTempErr_VentnActr19OvrTempErr_read/1 -> Terminator_480/1
  VentnActr19PsonSts_VentnActr19PsonSts_read/1 -> Terminator_481/1
  VentnActr19ResetSts_VentnActr19ResetSts_read/1 -> Terminator_482/1
  VentnActr19SpclFct_VentnActr19SpclFct_read/1 -> Terminator_483/1
  VentnActr19SpdSts_VentnActr19SpdSts_read/1 -> Terminator_484/1
  VentnActr19TrqSts_VentnActr19TrqSts_read/1 -> Terminator_485/1
  VentnActr19UrgcExist_VentnActr19UrgcExist_read/1 -> Terminator_486/1
  VentnActr19UrgcPson_VentnActr19UrgcPson_read/1 -> Terminator_487/1
  VentnActr19UrgcSts_VentnActr19UrgcSts_read/1 -> Terminator_488/1
  VentnActr19VoltErr_VentnActr19VoltErr_read/1 -> Terminator_489/1
  VentnActr20ActPson_VentnActr20ActPson_read/1 -> Terminator_490/1
  VentnActr20ActPson_VentnActr20ActPson_IsUpdated_read/1 -> Terminator_491/1
  VentnActr20BlckInd_VentnActr20BlckInd_read/1 -> Terminator_492/1
  VentnActr20BlckSts_VentnActr20BlckSts_read/1 -> Terminator_493/1
  VentnActr20BusAdr_VentnActr20BusAdr_read/1 -> Terminator_494/1
  VentnActr20CoilSts_VentnActr20CoilSts_read/1 -> Terminator_495/1
  VentnActr20DrvDir_VentnActr20DrvDir_read/1 -> Terminator_496/1
  VentnActr20ElecErr_VentnActr20ElecErr_read/1 -> Terminator_497/1
  VentnActr20Mode_VentnActr20Mode_read/1 -> Terminator_498/1
  VentnActr20MovDir_VentnActr20MovDir_read/1 -> Terminator_499/1
  VentnActr20OvrTempErr_VentnActr20OvrTempErr_read/1 -> Terminator_500/1
  VentnActr20PsonSts_VentnActr20PsonSts_read/1 -> Terminator_501/1
  VentnActr20ResetSts_VentnActr20ResetSts_read/1 -> Terminator_502/1
  VentnActr20SpclFct_VentnActr20SpclFct_read/1 -> Terminator_503/1
  VentnActr20SpdSts_VentnActr20SpdSts_read/1 -> Terminator_504/1
  VentnActr20TrqSts_VentnActr20TrqSts_read/1 -> Terminator_505/1
  VentnActr20UrgcExist_VentnActr20UrgcExist_read/1 -> Terminator_506/1
  VentnActr20UrgcPson_VentnActr20UrgcPson_read/1 -> Terminator_507/1
  VentnActr20UrgcSts_VentnActr20UrgcSts_read/1 -> Terminator_508/1
  VentnActr20VoltErr_VentnActr20VoltErr_read/1 -> Terminator_509/1
  VGAStopDuePowerLimit_VGAStopDuePowerLimit_read/1 -> Terminator_510/1
  BswLifeCycle_LifeCycle_read/1 -> Terminator_511/1
  DioServ_PowerOffInitChannel/1 -> Terminator_512/1
  DioServ_PowerOnInitChannel/1 -> Terminator_513/1
  Ground_1/1 -> DioServ_ReadChannel/1
  DioServ_ReadChannel/1 -> Terminator_514/1
  DioServ_ReadChannel/2 -> Terminator_515/1
  Ground_2/1 -> DioServ_WriteChannel/1
  Ground_3/1 -> DioServ_WriteChannel/2
  DioServ_WriteChannel/1 -> Terminator_516/1
  Ground_4/1 -> LinIfServ_GetScheduleInfo/1
  LinIfServ_GetScheduleInfo/1 -> Terminator_517/1
  LinIfServ_GetScheduleInfo/2 -> Terminator_518/1
  Ground_5/1 -> LinSMServ_ScheduleRequest/1
  Ground_6/1 -> LinSMServ_ScheduleRequest/2
  LinSMServ_ScheduleRequest/1 -> Terminator_519/1
  LinService_GetLin2WakeupStatus/1 -> Terminator_520/1
  LinService_GetLin2WakeupStatus/2 -> Terminator_521/1
  Ground_7/1 -> LinService_SendLin3dFrame/1
  Ground_8/1 -> LinService_SendLin3dFrame/2
  LinService_SendLin3dFrame/1 -> Terminator_522/1
  Ground_9/1 -> LinService_SetLin3cFrame/1
  Ground_10/1 -> LinService_SetLin3cFrame/2
  LinService_SetLin3cFrame/1 -> Terminator_523/1
  Ground_11/1 -> LinService_SetLinTable/1
  Ground_12/1 -> LinService_SetLinTable/2
  LinService_SetLinTable/1 -> Terminator_524/1
  MirrorLin3ErrServ_GetLinBusTxErrSta/1 -> Terminator_525/1
  MirrorLin3ErrServ_GetLinBusTxErrSta/2 -> Terminator_526/1
  Ground_13/1 -> MirrorLin3ErrServ_GetVgaCcmLin3VgaFr01NoResp/1
  MirrorLin3ErrServ_GetVgaCcmLin3VgaFr01NoResp/1 -> Terminator_527/1
  MirrorLin3ErrServ_GetVgaCcmLin3VgaFr01NoResp/2 -> Terminator_528/1
  Ground_14/1 -> MirrorLin3ErrServ_GetVgaRxErrSta/1
  MirrorLin3ErrServ_GetVgaRxErrSta/1 -> Terminator_529/1
  MirrorLin3ErrServ_GetVgaRxErrSta/2 -> Terminator_530/1
  Ground_15/1 -> MirrorLinFrameServ_GetRespSta/1
  MirrorLinFrameServ_GetRespSta/1 -> Terminator_531/1
  MirrorLinFrameServ_GetRespSta/2 -> Terminator_532/1
  MirrorLinFrameServ_GetRespSta/3 -> Terminator_533/1
  NvMServ_HfaMtrCalReadBlock/1 -> Terminator_534/1
  NvMServ_HfaMtrCalReadBlock/2 -> Terminator_535/1
  Ground_16/1 -> NvMServ_HfaMtrCalWriteBlock/1
  NvMServ_HfaMtrCalWriteBlock/1 -> Terminator_536/1
  Ground_17/1 -> NvMServ_NvMNotifyJobWorking/1
  NvMServ_NvMNotifyJobWorking/1 -> Terminator_537/1
  NvMServ_NvMNotifyJobWorking/2 -> Terminator_538/1
  NvMServ_VgaMtrCalReadBlock/1 -> Terminator_539/1
  NvMServ_VgaMtrCalReadBlock/2 -> Terminator_540/1
  Ground_18/1 -> NvMServ_VgaMtrCalWriteBlock/1
  NvMServ_VgaMtrCalWriteBlock/1 -> Terminator_541/1
  Ground_19/1 -> NvMServ_WriteBlock/1
  Ground_20/1 -> NvMServ_WriteBlock/2
  NvMServ_WriteBlock/1 -> Terminator_542/1
  PA_NvMBlockVgaMtrCalDataInit/1 -> Terminator_543/1
  Ground_21/1 -> PwrParaService_GetMotorCailCnt/1
  PwrParaService_GetMotorCailCnt/1 -> Terminator_544/1
  PwrParaService_GetMotorCailCnt/2 -> Terminator_545/1
  Ground_22/1 -> PwrParaService_GetMotorCailNum/1
  PwrParaService_GetMotorCailNum/1 -> Terminator_546/1
  PwrParaService_GetMotorCailNum/2 -> Terminator_547/1
  Ground_23/1 -> PwrParaService_SetMotorCailCnt/1
  Ground_24/1 -> PwrParaService_SetMotorCailCnt/2
  PwrParaService_SetMotorCailCnt/1 -> Terminator_548/1
  Ground_25/1 -> PwrParaService_SetMotorCailNum/1
  Ground_26/1 -> PwrParaService_SetMotorCailNum/2
  PwrParaService_SetMotorCailNum/1 -> Terminator_549/1
  Ground_27/1 -> VentnMotAdr_VentnMotAdr_write/1
  Ground_28/1 -> VentnMotAdr2_VentnMotAdr2_write/1
  Ground_29/1 -> VentnMotBlockCmd_VentnMotBlockCmd_write/1
  Ground_30/1 -> VentnMotBlockCmd2_VentnMotBlockCmd2_write/1
  Ground_31/1 -> VentnMotClearReq_VentnMotClearReq_write/1
  Ground_32/1 -> VentnMotClearReq2_VentnMotClearReq2_write/1
  Ground_33/1 -> VentnMotCoilPwrOn_VentnMotCoilPwrOn_write/1
  Ground_34/1 -> VentnMotCoilPwrOn2_VentnMotCoilPwrOn2_write/1
  Ground_35/1 -> VentnMotDir_VentnMotDir_write/1
  Ground_36/1 -> VentnMotDir2_VentnMotDir2_write/1
  Ground_37/1 -> VentnMotMode_VentnMotMode_write/1
  Ground_38/1 -> VentnMotMode2_VentnMotMode2_write/1
  Ground_39/1 -> VentnMotPsonAvl_VentnMotPsonAvl_write/1
  Ground_40/1 -> VentnMotPsonAvl2_VentnMotPsonAvl2_write/1
  Ground_41/1 -> VentnMotSaveData_VentnMotSaveData_write/1
  Ground_42/1 -> VentnMotSaveData2_VentnMotSaveData2_write/1
  Ground_43/1 -> VentnMotSpdSet_VentnMotSpdSet_write/1
  Ground_44/1 -> VentnMotSpdSet2_VentnMotSpdSet2_write/1
  Ground_45/1 -> VentnMotStrtPson_VentnMotStrtPson_write/1
  Ground_46/1 -> VentnMotStrtPson2_VentnMotStrtPson2_write/1
  Ground_47/1 -> VentnMotTargtPson_VentnMotTargtPson_write/1
  Ground_48/1 -> VentnMotTargtPson2_VentnMotTargtPson2_write/1
  Ground_49/1 -> VentnMotUrgcPson_VentnMotUrgcPson_write/1
  Ground_50/1 -> VentnMotUrgcPson2_VentnMotUrgcPson2_write/1
  Ground_51/1 -> VentnMotUrgcUnlck_VentnMotUrgcUnlck_write/1
  Ground_52/1 -> VentnMotUrgcUnlck2_VentnMotUrgcUnlck2_write/1
